simulink model "MCVT_Integration"  (R2020a)
settings: Solver=ode8  StartTime=0.0  StopTime=T_end  FixedStep=dt  RelTol=1e-3  EnableMultiTasking=on
values from neighbouring files (symbols this model uses):
  assumed_power_voltage = 1.25e2    (= 125)    [MCVT_Input_File.m]    (also in MCVT_Run_QuickCheck.m)
  battery_power_toggle = 1    [MCVT_Input_File.m]    (also in MCVT_Run_QuickCheck.m)
  battery_trickle_charge_param = 0.1    [MCVT_Input_File.m]    (also in MCVT_Run_QuickCheck.m)
  battery_trickle_leakage_param = 0.01    [MCVT_Input_File.m]    (also in MCVT_Run_QuickCheck.m)
  damage_case_id = 3    [MCVT_Input_File.m]    (also in MCVT_Run_QuickCheck.m = 1)
  dt = 1/fs    (= 0.001)    [MCVT_Run_QuickCheck.m]    (also in MCVT_Sim_Set.m)
  dt_2 = 1/fs_2    (= 0.001)    [MCVT_Run_QuickCheck.m]    (also in MCVT_Sim_Set.m)
  dt_3 = 1/fs_3    (= 0.001)    [MCVT_Run_QuickCheck.m]    (also in MCVT_Sim_Set.m)
  dt_5 = 1/fs_5    (= 0.005)    [MCVT_Run_QuickCheck.m]    (also in MCVT_Sim_Set.m)
  dt_8 = 1/fs_8    (= 0.05)    [MCVT_Run_QuickCheck.m]    (also in MCVT_Sim_Set.m)
  dt_9 = 1/fs_9    (= 0.001)    [MCVT_Run_QuickCheck.m]    (also in MCVT_Sim_Set.m)
  dust_met_duration = 3    [MCVT_Input_File.m]    (also in MCVT_Run_QuickCheck.m = 2)
  ECLSS_indoor_set_temperature = 298.15    [MCVT_Input_File.m]    (also in MCVT_Run_QuickCheck.m)
  energy_store_max_o = 150    [MCVT_Input_File.m]    (also in MCVT_Run_QuickCheck.m)
  energy_store_o = 120    [MCVT_Input_File.m]    (also in MCVT_Run_QuickCheck.m)
  fs = 1000    [MCVT_Run_QuickCheck.m]    (also in MCVT_Sim_Set.m)
  gain_charge_param = 1    [MCVT_Input_File.m]    (also in MCVT_Run_QuickCheck.m)
  ground_temperature = 373    [MCVT_Habitat_Design_Input_File.m]    (also in MCVT_Run_QuickCheck.m)
  I_max_charge = 800    [MCVT_Input_File.m]    (also in MCVT_Run_QuickCheck.m)
  I_max_discharge = 800    [MCVT_Input_File.m]    (also in MCVT_Run_QuickCheck.m)
  initial_pressure = 1e5    (= 100000)    [MCVT_Input_File.m]    (also in MCVT_Run_QuickCheck.m)
  initial_temperature = 295    [MCVT_Input_File.m]    (also in MCVT_Run_QuickCheck.m)
  interior_leak_rate = 0.00415    [MCVT_Input_File.m]    (also in MCVT_Run_QuickCheck.m)
  launch_dust_accum_start_time = 10    [MCVT_Input_File.m]    (also in MCVT_Run_QuickCheck.m = 2)
  launch_dust_accum_stop_time = 12    [MCVT_Input_File.m]    (also in MCVT_Run_QuickCheck.m = 3)
  meteorite_impact_time = 2    [MCVT_Input_File.m]    (also in MCVT_Run_QuickCheck.m = 1)
  nuclear_power_toggle = 1    [MCVT_Input_File.m]    (also in MCVT_Run_QuickCheck.m)
  print_interval = 30    [MCVT_Input_File.m]
  SOC_lower_thres = 0.5    [MCVT_Input_File.m]    (also in MCVT_Run_QuickCheck.m)
  SOC_upper_thres = 0.9    [MCVT_Input_File.m]    (also in MCVT_Run_QuickCheck.m)
  solar_power_toggle = 1    [MCVT_Input_File.m]    (also in MCVT_Run_QuickCheck.m)
  space_temperature = 4    [MCVT_Habitat_Design_Input_File.m]    (also in MCVT_Run_QuickCheck.m)
  Start_angle = 90    [MCVT_Input_File.m]    (also in MCVT_Run_QuickCheck.m)
undefined symbols (not defined in the file or in its folder): A_flow_restrictor, ACTIVITY_LOOKUP_TABLE, air, Aref, Bmap, Bmap2, Bmap3, comms, DiscIntNeverNeededParam, DmgSPL, dust_parameters, ECLSS_dust_damage_repair_time, ECLSS_heater_capacity, ECLSS_IPS_restrictor, ECLSS_IPS_setpointPressure, ECLSS_paint_damage_repair_time, ECLSS_radiatorLoop_discreteNumber, ECLSS_Vst, emptyActivity, expected_heat_add, FI, habitat, HIEM2SMM, HIEM2STM, HIEMDamageElements, impact_numbering, initial_SPLT_damage_index, input_air, input_Cond, input_CP, input_discretization, input_EV, input_Evap, input_radiatorLoop, K_ECLSS_dust_single, K_ECLSS_paint_single, K_Structure_damage_single, Kref, kW_to_W, meteoroidDustDep, mFRF, ndelays, nominalDustDep, noverlap, nuclear_toggle_gain, output_limits, panel_paint0, Ps0, reference_temperature, RepairTime_ECLSS_factor, Sensor_Location_SMM, SMM_T0, SMM_T1, SMM_T2, SMM_T3, SMM_T4, SMM_T4_0, SMM_T4_1, SMM_T4_2, solar_FDD_K ... (+73)
libraries: neural x350, simulink x39, xpcutilitieslib x7, xpcrtudplib x7, dspstat3 x1
(part 5 of 43)

== system MCVT_Integration/Sys3 - Power System/Power Generation Subsystem1/Power Distribution and Storage System/Subsystem2 ==
  Sub3_Power_Consumed_by_Sub2 (kW) : Inport
  Sub3_Power_Consumed_by_Sub5 (kW) : Inport  Port=2
  Sub3_Power_Consumed_by_Sub8 (kW) : Inport  Port=3
  2 : Memory  InitialCondition=50
  5 : Memory  InitialCondition=50
  Bus Creator1 : BusCreator  Inputs=3
  Dummy Electrical Power Consuming Equipment3 : Subsystem
  Sub3_Power_Concumption (kW) : Outport
  wires:
  Sub3_Power_Consumed_by_Sub5 (kW)/1 -> 5/1
  Sub3_Power_Consumed_by_Sub2 (kW)/1 -> 2/1
  Dummy Electrical Power Consuming Equipment3/1(p_3_desired) -> Bus Creator1/3  "p_3_desired"
  Bus Creator1/1 -> Sub3_Power_Concumption (kW)/1
  Sub3_Power_Consumed_by_Sub8 (kW)/1 -> Dummy Electrical Power Consuming Equipment3/1(XX_In_Power_Available)
  5/1 -> Bus Creator1/2  "p_2_desired"
  2/1 -> Bus Creator1/1  "p_1_desired"

== system MCVT_Integration/Sys5 - ECLSS/Active Thermal Control System (ATCS)/Faulty Panels/Sim-FDD-ECLSS-Dust ==
  xtrueHS : Inport
  EnableResetFDD_Dust : Inport  Port=2
  MATLAB Function2 : MATLAB Function  TreatAsAtomicUnit=on
  MATLAB System : MATLABSystem  System=ECLSS_simu_FDD_dust  timestep=[dt_5]  Ksingle=[K_ECLSS_dust_single]  noise=1e-2  num=50  SimulateUsing=Code generation
      mask Type: ECLSS_simu_FDD_dust
      mask Display:
      | disp('ECLSS_simu_FDD_dust');
      | port_label('input',1,'xtrue');
      | port_label('input',2,'EnableReset');
      | port_label('output',1,'xe');
  Scope : Scope  NumInputPorts=2
  FDD_ECLSS_dust_xe : Outport
  wires:
  EnableResetFDD_Dust/1 -> Scope/2, MATLAB System/2
  xtrueHS/1 -> MATLAB Function2/1(x)
  MATLAB Function2/1(y) -> MATLAB System/1
  MATLAB System/1 -> Scope/1, FDD_ECLSS_dust_xe/1

== system MCVT_Integration/Sys5 - ECLSS/Active Thermal Control System (ATCS)/Faulty Panels/Sim-FDD-ECLSS-Paint ==
  xtrueHS : Inport
  EnableResetFDD_Paint : Inport  Port=2
  MATLAB Function6 : MATLAB Function  TreatAsAtomicUnit=on
  MATLAB System1 : MATLABSystem  System=ECLSS_simu_FDD_paint  timestep=[dt_5]  Ksingle=[K_ECLSS_paint_single]  noise=1e-2  num=50  SimulateUsing=Code generation
      mask Type: ECLSS_simu_FDD_paint
      mask Display:
      | disp('ECLSS_simu_FDD_paint');
      | port_label('input',1,'xtrue');
      | port_label('input',2,'EnableReset');
      | port_label('output',1,'xe');
  FDD_ECLSS_paint_xe : Outport
  wires:
  MATLAB Function6/1(y) -> MATLAB System1/1
  EnableResetFDD_Paint/1 -> MATLAB System1/2
  MATLAB System1/1 -> FDD_ECLSS_paint_xe/1
  xtrueHS/1 -> MATLAB Function6/1(x)

== system MCVT_Integration/Sys8 - Interior Environment/Habitat Interior Environment Model/Temperature Sensors/ Resistance Temperature Detector ==
  Physical Interdependency - IN : Inport
  Switch Control : Inport  Port=2
  Power_Supply (kW) : Inport  Port=3
  Power Consumption : MATLAB Function  TreatAsAtomicUnit=on
  R = U/I : Gain  Gain=1/0.001
  R vs T : MATLAB Function  TreatAsAtomicUnit=on
  RTD with Self-heat Error : Subsystem
  RTD without Self-heat Error : Subsystem
  Self-heat Calculation : Subsystem
  Switch ON/OFF Control Block : Subsystem
  T vs R : MATLAB Function  TreatAsAtomicUnit=on
  Physical Interdependency - OUT : Outport  VectorParamsAs1DForOutWhenUnconnected=off
  Cyber Interdependency - OUT : Outport  Port=2  VectorParamsAs1DForOutWhenUnconnected=off
  Power_Usage (kW) : Outport  Port=3
  wires:
  T vs R/1(R) -> RTD without Self-heat Error/1(Resistance Change without Error), Self-heat Calculation/2(Resistance Change without Error)
  R vs T/1(T) -> Cyber Interdependency - OUT/1
  RTD with Self-heat Error/1(Voltage Output with Error) -> R = U/I/1
  Self-heat Calculation/1(Resistance Change with Error) -> RTD with Self-heat Error/1(Resistance Change with Error)
  R = U/I/1 -> R vs T/1(R)
  RTD without Self-heat Error/1(Current in Circuit) -> Self-heat Calculation/3(Current in Circuit)
  Switch ON/OFF Control Block/1(Out1) -> Power Consumption/2(Switch), T vs R/1(T), Self-heat Calculation/1(Real Temperature)
  Switch Control/1 -> Switch ON/OFF Control Block/1(In1)
  Physical Interdependency - IN/1 -> Physical Interdependency - OUT/1, Switch ON/OFF Control Block/2(In2)
  Power_Supply (kW)/1 -> Power Consumption/1(Supply)
  Power Consumption/1(kWUsage1) -> Power_Usage (kW)/1

== system MCVT_Integration/Sys8 - Interior Environment/Habitat Interior Environment Model/Temperature Sensors/ Resistance Temperature Detector1 ==
  Physical Interdependency - IN : Inport
  Switch Control : Inport  Port=2
  Power_Supply (kW) : Inport  Port=3
  Power Consumption : MATLAB Function  TreatAsAtomicUnit=on
  R = U/I : Gain  Gain=1/0.001
  R vs T : MATLAB Function  TreatAsAtomicUnit=on
  RTD with Self-heat Error : Subsystem
  RTD without Self-heat Error : Subsystem
  Self-heat Calculation : Subsystem
  Switch ON/OFF Control Block : Subsystem
  T vs R : MATLAB Function  TreatAsAtomicUnit=on
  Physical Interdependency - OUT : Outport  VectorParamsAs1DForOutWhenUnconnected=off
  Cyber Interdependency - OUT : Outport  Port=2  VectorParamsAs1DForOutWhenUnconnected=off
  Power_Usage (kW) : Outport  Port=3
  wires:
  Physical Interdependency - IN/1 -> Switch ON/OFF Control Block/2(In2), Physical Interdependency - OUT/1
  Switch Control/1 -> Switch ON/OFF Control Block/1(In1)
  RTD without Self-heat Error/1(Current in Circuit) -> Self-heat Calculation/3(Current in Circuit)
  R = U/I/1 -> R vs T/1(R)
  Self-heat Calculation/1(Resistance Change with Error) -> RTD with Self-heat Error/1(Resistance Change with Error)
  RTD with Self-heat Error/1(Voltage Output with Error) -> R = U/I/1
  R vs T/1(T) -> Cyber Interdependency - OUT/1
  T vs R/1(R) -> Self-heat Calculation/2(Resistance Change without Error), RTD without Self-heat Error/1(Resistance Change without Error)
  Power Consumption/1(kWUsage2) -> Power_Usage (kW)/1
  Switch ON/OFF Control Block/1(Out1) -> Power Consumption/2(Switch), Self-heat Calculation/1(Real Temperature), T vs R/1(T)
  Power_Supply (kW)/1 -> Power Consumption/1(Supply)

== system MCVT_Integration/Sys8 - Interior Environment/Habitat Interior Environment Model/Temperature Sensors/ Resistance Temperature Detector2 ==
  Physical Interdependency - IN : Inport
  Switch Control : Inport  Port=2
  Power_Supply (kW) : Inport  Port=3
  Power Consumption : MATLAB Function  TreatAsAtomicUnit=on
  R = U/I : Gain  Gain=1/0.001
  R vs T : MATLAB Function  TreatAsAtomicUnit=on
  RTD with Self-heat Error : Subsystem
  RTD without Self-heat Error : Subsystem
  Self-heat Calculation : Subsystem
  Switch ON/OFF Control Block : Subsystem
  T vs R : MATLAB Function  TreatAsAtomicUnit=on
  Physical Interdependency - OUT : Outport  VectorParamsAs1DForOutWhenUnconnected=off
  Cyber Interdependency - OUT : Outport  Port=2  VectorParamsAs1DForOutWhenUnconnected=off
  Power_Usage (kW) : Outport  Port=3
  wires:
  T vs R/1(R) -> RTD without Self-heat Error/1(Resistance Change without Error), Self-heat Calculation/2(Resistance Change without Error)
  R vs T/1(T) -> Cyber Interdependency - OUT/1
  RTD with Self-heat Error/1(Voltage Output with Error) -> R = U/I/1
  Self-heat Calculation/1(Resistance Change with Error) -> RTD with Self-heat Error/1(Resistance Change with Error)
  R = U/I/1 -> R vs T/1(R)
  RTD without Self-heat Error/1(Current in Circuit) -> Self-heat Calculation/3(Current in Circuit)
  Switch Control/1 -> Switch ON/OFF Control Block/1(In1)
  Physical Interdependency - IN/1 -> Switch ON/OFF Control Block/2(In2), Physical Interdependency - OUT/1
  Power Consumption/1(kWUsage3) -> Power_Usage (kW)/1
  Switch ON/OFF Control Block/1(Out1) -> Power Consumption/2(Switch), T vs R/1(T), Self-heat Calculation/1(Real Temperature)
  Power_Supply (kW)/1 -> Power Consumption/1(Supply)

== system MCVT_Integration/Sys 6 - Health Management and Agents/Decision Maker/Simulink Communication Modules/Agent//MCVT API from HMS/UDP_Receive_C2 ==
  "Byte Unpacking " : xpcutilitieslib/Byte Unpacking   MaskUnpackedDataTypes={'uint8','uint16','double'}  MaskUnpackedDataSizes={[6],[1],[comms.recv.Agent.nD1]}  MaskAlignment=1
      settings: SourceProductBaseCode=XP
  Data Type Conversion : DataTypeConversion  OutDataTypeStr=double
  Mux : Mux  Inputs=2
  Receive : xpcrtudplib/UDP Receive  ipAddressVal=Use host-target connection  localPort=comms.recv.Agent.LPORT  rcvWidth=comms.recv.Agent.nD1*8+8  rcvFmAny=off  fmAddress=comms.Remote_IP  rcvMulticast=off  multicastAddress={}  maxUDPQueue=8  sampleTime=dt_2
  Terminator : Terminator
  Receive_file : Outport
  Receive_output : Outport  Port=2
  Receive_length : Outport  Port=3
  wires:
  Receive/1 -> "Byte Unpacking "/1
  "Byte Unpacking "/3 -> Mux/1, Receive_output/1
  "Byte Unpacking "/1 -> Terminator/1
  Receive/2 -> Receive_length/1
  Mux/1 -> Receive_file/1
  "Byte Unpacking "/2 -> Data Type Conversion/1
  Data Type Conversion/1 -> Mux/2

== system MCVT_Integration/Sys 6 - Health Management and Agents/Decision Maker/Simulink Communication Modules/Agent//MCVT API to HMS/UDP_Send_C2 ==
  Input_Send : Inport
  Packet_Info : Inport  Port=2
  "Byte Packing " : xpcutilitieslib/Byte Packing   MaskPackedDataType=uint8  MaskUnpackedDataTypes={'uint8','uint16','double'}  MaskAlignment=1
  Data Type Conversion : DataTypeConversion  OutDataTypeStr=uint8
  Data Type Conversion1 : DataTypeConversion  OutDataTypeStr=uint16
  MATLAB Function : MATLAB Function  TreatAsAtomicUnit=on
  MATLAB Function1 : MATLAB Function  TreatAsAtomicUnit=on
  Send1 : xpcrtudplib/UDP Send  ipAddressVal=Use host-target connection  localPort=comms.send.Agent.LPORT  toAddress=comms.Remote_IP  toPort=comms.send.Agent.TOPORT  sampleTime=comms.dt_NRT_comm
      settings: SourceProductBaseCode=XP
  Width : Width
  Width1 : Width
  Send_file : Outport
  wires:
  Width1/1 -> MATLAB Function/1(len)
  Input_Send/1 -> Send_file/1, "Byte Packing "/3, Width1/1, MATLAB Function1/1(signal)
  "Byte Packing "/1 -> Width/1, Send1/1
  Width/1 -> Send1/2
  MATLAB Function/1(hi0) -> Data Type Conversion/1
  Data Type Conversion/1 -> "Byte Packing "/1
  Packet_Info/1 -> MATLAB Function/2(hdr_Info)
  MATLAB Function1/1(SizeInfo) -> MATLAB Function/3(rowcol)
  MATLAB Function/2(len0) -> Data Type Conversion1/1
  Data Type Conversion1/1 -> "Byte Packing "/2

== system MCVT_Integration/Sys 6 - Health Management and Agents/Decision Maker/Simulink Communication Modules/ECLSS_Dust//MCVT API to HMS/UDP_Send ==
  Input_Send : Inport
  Packet_Info : Inport  Port=2
  "Byte Packing " : xpcutilitieslib/Byte Packing   MaskPackedDataType=uint8  MaskUnpackedDataTypes={'uint8','uint16','double'}  MaskAlignment=1
  Data Type Conversion : DataTypeConversion  OutDataTypeStr=uint8
  Data Type Conversion1 : DataTypeConversion  OutDataTypeStr=uint16
  MATLAB Function : MATLAB Function  TreatAsAtomicUnit=on
  MATLAB Function1 : MATLAB Function  TreatAsAtomicUnit=on
  Send1 : xpcrtudplib/UDP Send  ipAddressVal=Use host-target connection  localPort=comms.send.ECLSS.FDD_Dust.LPORT  toAddress=comms.Remote_IP  toPort=comms.send.ECLSS.FDD_Dust.TOPORT  sampleTime=comms.dt_NRT_comm
      settings: SourceProductBaseCode=XP
  Width : Width
  Width1 : Width
  Send_file : Outport
  wires:
  Width1/1 -> MATLAB Function/1(len)
  Input_Send/1 -> Send_file/1, "Byte Packing "/3, Width1/1, MATLAB Function1/1(signal)
  "Byte Packing "/1 -> Width/1, Send1/1
  Width/1 -> Send1/2
  MATLAB Function/1(hi0) -> Data Type Conversion/1
  Data Type Conversion/1 -> "Byte Packing "/1
  MATLAB Function1/1(SizeInfo) -> MATLAB Function/3(rowcol)
  MATLAB Function/2(len0) -> Data Type Conversion1/1
  Data Type Conversion1/1 -> "Byte Packing "/2
  Packet_Info/1 -> MATLAB Function/2(hdr_Info)

== system MCVT_Integration/Sys 6 - Health Management and Agents/Decision Maker/Simulink Communication Modules/ECLSS_Paint//MCVT API to HMS/UDP_Send ==
  Input_Send : Inport
  Packet_Info : Inport  Port=2
  "Byte Packing " : xpcutilitieslib/Byte Packing   MaskPackedDataType=uint8  MaskUnpackedDataTypes={'uint8','uint16','double'}  MaskAlignment=1
  Data Type Conversion : DataTypeConversion  OutDataTypeStr=uint8
  Data Type Conversion1 : DataTypeConversion  OutDataTypeStr=uint16
  MATLAB Function : MATLAB Function  TreatAsAtomicUnit=on
  MATLAB Function1 : MATLAB Function  TreatAsAtomicUnit=on
  Send1 : xpcrtudplib/UDP Send  ipAddressVal=Use host-target connection  localPort=comms.send.ECLSS.FDD_Paint.LPORT  toAddress=comms.Remote_IP  toPort=comms.send.ECLSS.FDD_Paint.TOPORT  sampleTime=comms.dt_NRT_comm
      settings: SourceProductBaseCode=XP
  Width : Width
  Width1 : Width
  Send_file : Outport
  wires:
  Width1/1 -> MATLAB Function/1(len)
  Input_Send/1 -> Send_file/1, "Byte Packing "/3, Width1/1, MATLAB Function1/1(signal)
  "Byte Packing "/1 -> Width/1, Send1/1
  Width/1 -> Send1/2
  MATLAB Function/1(hi0) -> Data Type Conversion/1
  Data Type Conversion/1 -> "Byte Packing "/1
  Packet_Info/1 -> MATLAB Function/2(hdr_Info)
  MATLAB Function1/1(SizeInfo) -> MATLAB Function/3(rowcol)
  MATLAB Function/2(len0) -> Data Type Conversion1/1
  Data Type Conversion1/1 -> "Byte Packing "/2

== system MCVT_Integration/Sys 6 - Health Management and Agents/Decision Maker/Simulink Communication Modules/NPG_Dust//MCVT API to HMS/UDP_Send ==
  Input_Send : Inport
  Packet_Info : Inport  Port=2
  "Byte Packing " : xpcutilitieslib/Byte Packing   MaskPackedDataType=uint8  MaskUnpackedDataTypes={'uint8','uint16','double'}  MaskAlignment=1
  Data Type Conversion : DataTypeConversion  OutDataTypeStr=uint8
  Data Type Conversion1 : DataTypeConversion  OutDataTypeStr=uint16
  MATLAB Function : MATLAB Function  TreatAsAtomicUnit=on
  MATLAB Function1 : MATLAB Function  TreatAsAtomicUnit=on
  Send1 : xpcrtudplib/UDP Send  ipAddressVal=Use host-target connection  localPort=comms.send.NPG.FDD_Dust.LPORT  toAddress=comms.Remote_IP  toPort=comms.send.NPG.FDD_Dust.TOPORT  sampleTime=comms.dt_NRT_comm
      settings: SourceProductBaseCode=XP
  Width : Width
  Width1 : Width
  Send_file : Outport
  wires:
  Width1/1 -> MATLAB Function/1(len)
  Input_Send/1 -> Send_file/1, "Byte Packing "/3, Width1/1, MATLAB Function1/1(signal)
  "Byte Packing "/1 -> Width/1, Send1/1
  Width/1 -> Send1/2
  MATLAB Function/1(hi0) -> Data Type Conversion/1
  Data Type Conversion/1 -> "Byte Packing "/1
  Packet_Info/1 -> MATLAB Function/2(hdr_Info)
  MATLAB Function1/1(SizeInfo) -> MATLAB Function/3(rowcol)
  MATLAB Function/2(len0) -> Data Type Conversion1/1
  Data Type Conversion1/1 -> "Byte Packing "/2

== system MCVT_Integration/Sys 6 - Health Management and Agents/Decision Maker/Simulink Communication Modules/Solar_PV//MCVT API to HMS/UDP_Send ==
  Input_Send : Inport
  Packet_Info : Inport  Port=2
  "Byte Packing " : xpcutilitieslib/Byte Packing   MaskPackedDataType=uint8  MaskUnpackedDataTypes={'uint8','uint16','double'}  MaskAlignment=1
  Data Type Conversion : DataTypeConversion  OutDataTypeStr=uint8
  Data Type Conversion1 : DataTypeConversion  OutDataTypeStr=uint16
  MATLAB Function : MATLAB Function  TreatAsAtomicUnit=on
  MATLAB Function1 : MATLAB Function  TreatAsAtomicUnit=on
  Send1 : xpcrtudplib/UDP Send  ipAddressVal=Use host-target connection  localPort=comms.send.SPG.FDD_Dust.LPORT  toAddress=comms.Remote_IP  toPort=comms.send.SPG.FDD_Dust.TOPORT  sampleTime=comms.dt_NRT_comm
      settings: SourceProductBaseCode=XP
  Width : Width
  Width1 : Width
  Send_file : Outport
  wires:
  Width1/1 -> MATLAB Function/1(len)
  Input_Send/1 -> Send_file/1, "Byte Packing "/3, Width1/1, MATLAB Function1/1(signal)
  "Byte Packing "/1 -> Width/1, Send1/1
  Width/1 -> Send1/2
  MATLAB Function/1(hi0) -> Data Type Conversion/1
  Data Type Conversion/1 -> "Byte Packing "/1
  Packet_Info/1 -> MATLAB Function/2(hdr_Info)
  MATLAB Function1/1(SizeInfo) -> MATLAB Function/3(rowcol)
  MATLAB Function/2(len0) -> Data Type Conversion1/1
  Data Type Conversion1/1 -> "Byte Packing "/2

== system MCVT_Integration/Sys 6 - Health Management and Agents/Decision Maker/Simulink Communication Modules/Structure_Dmg//MCVT API to HMS/UDP_Send ==
  Input_Send : Inport
  Packet_Info : Inport  Port=2
  "Byte Packing " : xpcutilitieslib/Byte Packing   MaskPackedDataType=uint8  MaskUnpackedDataTypes={'uint8','uint16','double'}  MaskAlignment=1
  Data Type Conversion : DataTypeConversion  OutDataTypeStr=uint8
  Data Type Conversion1 : DataTypeConversion  OutDataTypeStr=uint16
  MATLAB Function : MATLAB Function  TreatAsAtomicUnit=on
  MATLAB Function1 : MATLAB Function  TreatAsAtomicUnit=on
  Send1 : xpcrtudplib/UDP Send  ipAddressVal=Use host-target connection  localPort=comms.send.Str.FDD_Dmg.LPORT  toAddress=comms.Remote_IP  toPort=comms.send.Str.FDD_Dmg.TOPORT  sampleTime=comms.dt_NRT_comm
      settings: SourceProductBaseCode=XP
  Width : Width
  Width1 : Width
  Send_file : Outport
  wires:
  Width1/1 -> MATLAB Function/1(len)
  Input_Send/1 -> Send_file/1, "Byte Packing "/3, Width1/1, MATLAB Function1/1(signal)
  "Byte Packing "/1 -> Width/1, Send1/1
  Width/1 -> Send1/2
  MATLAB Function/1(hi0) -> Data Type Conversion/1
  Data Type Conversion/1 -> "Byte Packing "/1
  Packet_Info/1 -> MATLAB Function/2(hdr_Info)
  MATLAB Function1/1(SizeInfo) -> MATLAB Function/3(rowcol)
  MATLAB Function/2(len0) -> Data Type Conversion1/1
  Data Type Conversion1/1 -> "Byte Packing "/2

== system MCVT_Integration/Sys2 - Structural System/Sys2_Structural_System/Fault Detection and Diagnosis (FDD) 2.3/Accelerometer/Acceleration Delay ==
  Measured Acceleration : Inport
  Matrix Concatenate : Concatenate  NumInputs=9  Mode=Multidimensional array  ConcatenateDimension=2
  Selector1 : Selector  InputPortWidth=9  IndexOptions=Index vector (dialog)  Indices=[1]  OutputSizes=1
  Selector2 : Selector  InputPortWidth=9  IndexOptions=Index vector (dialog)  Indices=[2]  OutputSizes=1
  Selector3 : Selector  InputPortWidth=9  IndexOptions=Index vector (dialog)  Indices=[3]  OutputSizes=1
  Selector4 : Selector  InputPortWidth=9  IndexOptions=Index vector (dialog)  Indices=[4]  OutputSizes=1
  Selector5 : Selector  InputPortWidth=9  IndexOptions=Index vector (dialog)  Indices=[5]  OutputSizes=1
  Selector6 : Selector  InputPortWidth=9  IndexOptions=Index vector (dialog)  Indices=[6]  OutputSizes=1
  Selector7 : Selector  InputPortWidth=9  IndexOptions=Index vector (dialog)  Indices=[7]  OutputSizes=1
  Selector8 : Selector  InputPortWidth=9  IndexOptions=Index vector (dialog)  Indices=[8]  OutputSizes=1
  Selector9 : Selector  InputPortWidth=9  IndexOptions=Index vector (dialog)  Indices=[9]  OutputSizes=1
  Tapped Delay {1..9} (9 alike) : simulink/Discrete/Tapped Delay  vinit=0.0  samptime=-1  NumDelays=ndelays  DelayOrder=Oldest  includeCurrent=off
  Delayed Acceleration : Outport
  wires:
  Tapped Delay 9/1 -> Matrix Concatenate/9
  Tapped Delay 8/1 -> Matrix Concatenate/8
  Tapped Delay 7/1 -> Matrix Concatenate/7
  Tapped Delay 6/1 -> Matrix Concatenate/6
  Tapped Delay 5/1 -> Matrix Concatenate/5
  Tapped Delay 4/1 -> Matrix Concatenate/4
  Tapped Delay 3/1 -> Matrix Concatenate/3
  Tapped Delay 2/1 -> Matrix Concatenate/2
  Tapped Delay 1/1 -> Matrix Concatenate/1
  Measured Acceleration/1 -> Selector1/1, Selector2/1, Selector3/1, Selector4/1, Selector5/1, Selector6/1, Selector7/1, Selector8/1, Selector9/1
  Selector2/1 -> Tapped Delay 2/1  "Actural Acceleration"
  Selector1/1 -> Tapped Delay 1/1  "Actural Acceleration"
  Selector7/1 -> Tapped Delay 7/1  "Actural Acceleration"
  Selector6/1 -> Tapped Delay 6/1  "Actural Acceleration"
  Selector4/1 -> Tapped Delay 4/1  "Actural Acceleration"
  Selector5/1 -> Tapped Delay 5/1  "Actural Acceleration"
  Selector3/1 -> Tapped Delay 3/1  "Actural Acceleration"
  Matrix Concatenate/1 -> Delayed Acceleration/1
  Selector9/1 -> Tapped Delay 9/1  "Actural Acceleration"
  Selector8/1 -> Tapped Delay 8/1  "Actural Acceleration"

== system MCVT_Integration/Sys2 - Structural System/Sys2_Structural_System/Fault Detection and Diagnosis (FDD) 2.3/Accelerometer/Accelerometer ==
  note: 1 -- Turn sensors on
      | 2 -- Turn sesnors off
  note: Damage States
      | State 1 - Healthy
      | State 2 - Partially Damaged1: Amplitude Change
      | State 3 - Partially Damaged2: Data Loss
      | State 4 - Failed
  Physical Interdependency - IN : Inport
  SensorPowerSupply : Inport  Port=2
  " Switch ON/OFF Control Number" : MATLAB Function  TreatAsAtomicUnit=on
  Accelerometer : Subsystem
  Bias Voltage : Constant  Value=12
  Clock : Clock
  Damage State Control Block : MATLAB Function  TreatAsAtomicUnit=on
  Damage State Control Number : Constant  Value=[1;1;1]
  Minus : Sum  Inputs=+-
  "Sensitivity " : Gain  Gain=1000
  Sensor Number : Constant  Value=[1;2;3]
  Switch ON/OFF Control Block : Subsystem
  Cyber Interdependency - OUT : Outport  VectorParamsAs1DForOutWhenUnconnected=off
  wires:
  "Sensitivity "/1 -> Cyber Interdependency - OUT/1
  Accelerometer/1(Out) -> Damage State Control Block/2(Acc)
  Damage State Control Block/1(y) -> Minus/1
  Damage State Control Number/1 -> Damage State Control Block/1(state_number)
  Clock/1 -> Damage State Control Block/3(Time)
  Sensor Number/1 -> Switch ON/OFF Control Block/2(In2)
  Switch ON/OFF Control Block/1(Out1) -> Accelerometer/1(In1)
  Physical Interdependency - IN/1 -> Switch ON/OFF Control Block/3(In3)
  Bias Voltage/1 -> Minus/2
  Minus/1 -> "Sensitivity "/1
  SensorPowerSupply/1 -> " Switch ON/OFF Control Number"/1(p)
  " Switch ON/OFF Control Number"/1(y) -> Switch ON/OFF Control Block/1(In1)

== system MCVT_Integration/Sys2 - Structural System/Sys2_Structural_System/Fault Detection and Diagnosis (FDD) 2.3/Pattern Recognition Neural Network/Pattern Recognition Neural Network with 10% Noise ==
  Input : Inport  PortDimensions=2304
      mask Display: e = 0.06;c1 = [120 230 180]/255;c2 = ([120 230 180]+0)/(256+128);c3 = ([120 230 180]+128)/(256+128);patch([0 1 1 0],[0 0 1 1],c1);patch([0 1 1 1-e 1-e 0+e],[0 0 1 1-e 0+e 0+e],c2);patch([0 0 1 1-e 0+e 0+e],[0 1 1 1-e 1-e 0+e],c3);plot([0 1 1 0 0],[0 0 1 1 0]);
  Layer 1 : Subsystem
      mask Display: e = 0.06;c1 = [120 150 230]/255;c2 = ([120 150 230]+0)/383;c3 = ([120 150 230]+128)/383;patch([0 1 1 0],[0 0 1 1],c1);patch([0 1 1 1-e 1-e 0+e],[0 0 1 1-e 0+e 0+e],c2);patch([0 0 1 1-e 0+e 0+e],[0 1 1 1-e 1-e 0+e],c3);plot([0 1 1 0 0],[0 0 1 1 0]);
  Layer 2 : Subsystem
      mask Display: e = 0.06;c1 = [120 150 230]/255;c2 = ([120 150 230]+0)/383;c3 = ([120 150 230]+128)/383;patch([0 1 1 0],[0 0 1 1],c1);patch([0 1 1 1-e 1-e 0+e],[0 0 1 1-e 0+e 0+e],c2);patch([0 0 1 1-e 0+e 0+e],[0 1 1 1-e 1-e 0+e],c3);plot([0 1 1 0 0],[0 0 1 1 0]);
  Layer 3 : Subsystem
      mask Display: e = 0.06;c1 = [120 150 230]/255;c2 = ([120 150 230]+0)/383;c3 = ([120 150 230]+128)/383;patch([0 1 1 0],[0 0 1 1],c1);patch([0 1 1 1-e 1-e 0+e],[0 0 1 1-e 0+e 0+e],c2);patch([0 0 1 1-e 0+e 0+e],[0 1 1 1-e 1-e 0+e],c3);plot([0 1 1 0 0],[0 0 1 1 0]);
  Process Input 1 : Subsystem
      mask Display: e = 0.06;c1 = [120 150 230]/255;c2 = ([120 150 230]+0)/383;c3 = ([120 150 230]+128)/383;patch([0 1 1 0],[0 0 1 1],c1);patch([0 1 1 1-e 1-e 0+e],[0 0 1 1-e 0+e 0+e],c2);patch([0 0 1 1-e 0+e 0+e],[0 1 1 1-e 1-e 0+e],c3);plot([0 1 1 0 0],[0 0 1 1 0]);
  Process Output 1 : Subsystem
      mask Display: e = 0.06;c1 = [120 150 230]/255;c2 = ([120 150 230]+0)/383;c3 = ([120 150 230]+128)/383;patch([0 1 1 0],[0 0 1 1],c1);patch([0 1 1 1-e 1-e 0+e],[0 0 1 1-e 0+e 0+e],c2);patch([0 0 1 1-e 0+e 0+e],[0 1 1 1-e 1-e 0+e],c3);plot([0 1 1 0 0],[0 0 1 1 0]);
  Output : Outport  InitialOutput=[0;0;0;0;0;0]
      mask Display: e = 0.06;c1 = [120 230 180]/255;c2 = ([120 230 180]+0)/(256+128);c3 = ([120 230 180]+128)/(256+128);patch([0 1 1 0],[0 0 1 1],c1);patch([0 1 1 1-e 1-e 0+e],[0 0 1 1-e 0+e 0+e],c2);patch([0 0 1 1-e 0+e 0+e],[0 1 1 1-e 1-e 0+e],c3);plot([0 1 1 0 0],[0 0 1 1 0]);
  wires:
  Input/1 -> Process Input 1/1(x)
  Process Output 1/1(y) -> Output/1
  Process Input 1/1(p) -> Layer 1/1("p{1}")
  Layer 1/1("a{1}") -> [feedback1]
  [feedback1] -> Layer 2/1("a{1} ")
  Layer 2/1("a{2}") -> [feedback2]
  [feedback2] -> Layer 3/1("a{2} ")
  Layer 3/1("a{3}") -> Process Output 1/1(a)

== system MCVT_Integration/Sys2 - Structural System/Sys2_Structural_System/Fault Detection and Diagnosis (FDD) with Binary Classifer/Accelerometer/Acceleration Delay ==
  Measured Acceleration : Inport
  Matrix Concatenate : Concatenate  NumInputs=9  Mode=Multidimensional array  ConcatenateDimension=2
  Selector1 : Selector  InputPortWidth=9  IndexOptions=Index vector (dialog)  Indices=[1]  OutputSizes=1
  Selector2 : Selector  InputPortWidth=9  IndexOptions=Index vector (dialog)  Indices=[2]  OutputSizes=1
  Selector3 : Selector  InputPortWidth=9  IndexOptions=Index vector (dialog)  Indices=[3]  OutputSizes=1
  Selector4 : Selector  InputPortWidth=9  IndexOptions=Index vector (dialog)  Indices=[4]  OutputSizes=1
  Selector5 : Selector  InputPortWidth=9  IndexOptions=Index vector (dialog)  Indices=[5]  OutputSizes=1
  Selector6 : Selector  InputPortWidth=9  IndexOptions=Index vector (dialog)  Indices=[6]  OutputSizes=1
  Selector7 : Selector  InputPortWidth=9  IndexOptions=Index vector (dialog)  Indices=[7]  OutputSizes=1
  Selector8 : Selector  InputPortWidth=9  IndexOptions=Index vector (dialog)  Indices=[8]  OutputSizes=1
  Selector9 : Selector  InputPortWidth=9  IndexOptions=Index vector (dialog)  Indices=[9]  OutputSizes=1
  Tapped Delay {1..9} (9 alike) : simulink/Discrete/Tapped Delay  vinit=0.0  samptime=-1  NumDelays=ndelays  DelayOrder=Oldest  includeCurrent=off
  Delayed Acceleration : Outport
  wires:
  Tapped Delay 9/1 -> Matrix Concatenate/9
  Tapped Delay 8/1 -> Matrix Concatenate/8
  Tapped Delay 7/1 -> Matrix Concatenate/7
  Tapped Delay 6/1 -> Matrix Concatenate/6
  Tapped Delay 5/1 -> Matrix Concatenate/5
  Tapped Delay 4/1 -> Matrix Concatenate/4
  Tapped Delay 3/1 -> Matrix Concatenate/3
  Tapped Delay 2/1 -> Matrix Concatenate/2
  Tapped Delay 1/1 -> Matrix Concatenate/1
  Measured Acceleration/1 -> Selector1/1, Selector2/1, Selector3/1, Selector4/1, Selector5/1, Selector6/1, Selector7/1, Selector8/1, Selector9/1
  Selector2/1 -> Tapped Delay 2/1  "Actural Acceleration"
  Selector1/1 -> Tapped Delay 1/1  "Actural Acceleration"
  Selector7/1 -> Tapped Delay 7/1  "Actural Acceleration"
  Selector6/1 -> Tapped Delay 6/1  "Actural Acceleration"
  Selector4/1 -> Tapped Delay 4/1  "Actural Acceleration"
  Selector5/1 -> Tapped Delay 5/1  "Actural Acceleration"
  Selector3/1 -> Tapped Delay 3/1  "Actural Acceleration"
  Matrix Concatenate/1 -> Delayed Acceleration/1
  Selector9/1 -> Tapped Delay 9/1  "Actural Acceleration"
  Selector8/1 -> Tapped Delay 8/1  "Actural Acceleration"

== system MCVT_Integration/Sys2 - Structural System/Sys2_Structural_System/Fault Detection and Diagnosis (FDD) with Binary Classifer/Accelerometer/Accelerometer ==
  note: 1 -- Turn sensors on
      | 2 -- Turn sesnors off
  note: Damage States
      | State 1 - Healthy
      | State 2 - Partially Damaged1: Amplitude Change
      | State 3 - Partially Damaged2: Data Loss
      | State 4 - Totally Failed
  Physical Interdependency - IN : Inport
  SensorPowerSupply : Inport  Port=2
  " Switch ON/OFF Control Number" : MATLAB Function  TreatAsAtomicUnit=on
  Accelerometer : Subsystem
  Actural Acc : Scope
  Bias Voltage : Constant  Value=12
  Clock : Clock
  Damage State Control Block : MATLAB Function  TreatAsAtomicUnit=on
  Damage State Control Number : Constant  Value=[1;1;1]
  Measured Acc : Scope
  Minus : Sum  Inputs=+-
  "Sensitivity " : Gain  Gain=1000
  Sensor Number : Constant  Value=[1;2;3]
  Switch ON/OFF Control Block : Subsystem
  Cyber Interdependency - OUT : Outport  VectorParamsAs1DForOutWhenUnconnected=off
  wires:
  "Sensitivity "/1 -> Cyber Interdependency - OUT/1, Measured Acc/1
  Accelerometer/1(Out) -> Damage State Control Block/2(Acc)
  Damage State Control Block/1(y) -> Minus/1
  Damage State Control Number/1 -> Damage State Control Block/1(state_number)
  Clock/1 -> Damage State Control Block/3(Time)
  Sensor Number/1 -> Switch ON/OFF Control Block/2(In2)
  Switch ON/OFF Control Block/1(Out1) -> Accelerometer/1(In1)
  Physical Interdependency - IN/1 -> Actural Acc/1, Switch ON/OFF Control Block/3(In3)
  Bias Voltage/1 -> Minus/2
  Minus/1 -> "Sensitivity "/1
  SensorPowerSupply/1 -> " Switch ON/OFF Control Number"/1(p)
  " Switch ON/OFF Control Number"/1(y) -> Switch ON/OFF Control Block/1(In1)

== system MCVT_Integration/Sys2 - Structural System/Sys2_Structural_System/Fault Detection and Diagnosis (FDD) with Binary Classifer/Pattern Recognition Neural Network/Segment 1 ==
  Input : Inport  PortDimensions=2304
      mask Display: e = 0.06;c1 = [120 230 180]/255;c2 = ([120 230 180]+0)/(256+128);c3 = ([120 230 180]+128)/(256+128);patch([0 1 1 0],[0 0 1 1],c1);patch([0 1 1 1-e 1-e 0+e],[0 0 1 1-e 0+e 0+e],c2);patch([0 0 1 1-e 0+e 0+e],[0 1 1 1-e 1-e 0+e],c3);plot([0 1 1 0 0],[0 0 1 1 0]);
  Layer 1 : Subsystem
      mask Display: e = 0.06;c1 = [120 150 230]/255;c2 = ([120 150 230]+0)/383;c3 = ([120 150 230]+128)/383;patch([0 1 1 0],[0 0 1 1],c1);patch([0 1 1 1-e 1-e 0+e],[0 0 1 1-e 0+e 0+e],c2);patch([0 0 1 1-e 0+e 0+e],[0 1 1 1-e 1-e 0+e],c3);plot([0 1 1 0 0],[0 0 1 1 0]);
  Layer 2 : Subsystem
      mask Display: e = 0.06;c1 = [120 150 230]/255;c2 = ([120 150 230]+0)/383;c3 = ([120 150 230]+128)/383;patch([0 1 1 0],[0 0 1 1],c1);patch([0 1 1 1-e 1-e 0+e],[0 0 1 1-e 0+e 0+e],c2);patch([0 0 1 1-e 0+e 0+e],[0 1 1 1-e 1-e 0+e],c3);plot([0 1 1 0 0],[0 0 1 1 0]);
  Layer 3 : Subsystem
      mask Display: e = 0.06;c1 = [120 150 230]/255;c2 = ([120 150 230]+0)/383;c3 = ([120 150 230]+128)/383;patch([0 1 1 0],[0 0 1 1],c1);patch([0 1 1 1-e 1-e 0+e],[0 0 1 1-e 0+e 0+e],c2);patch([0 0 1 1-e 0+e 0+e],[0 1 1 1-e 1-e 0+e],c3);plot([0 1 1 0 0],[0 0 1 1 0]);
  Process Input 1 : Subsystem
      mask Display: e = 0.06;c1 = [120 150 230]/255;c2 = ([120 150 230]+0)/383;c3 = ([120 150 230]+128)/383;patch([0 1 1 0],[0 0 1 1],c1);patch([0 1 1 1-e 1-e 0+e],[0 0 1 1-e 0+e 0+e],c2);patch([0 0 1 1-e 0+e 0+e],[0 1 1 1-e 1-e 0+e],c3);plot([0 1 1 0 0],[0 0 1 1 0]);
  Process Output 1 : Subsystem
      mask Display: e = 0.06;c1 = [120 150 230]/255;c2 = ([120 150 230]+0)/383;c3 = ([120 150 230]+128)/383;patch([0 1 1 0],[0 0 1 1],c1);patch([0 1 1 1-e 1-e 0+e],[0 0 1 1-e 0+e 0+e],c2);patch([0 0 1 1-e 0+e 0+e],[0 1 1 1-e 1-e 0+e],c3);plot([0 1 1 0 0],[0 0 1 1 0]);
  Output : Outport  InitialOutput=[0;0]
      mask Display: e = 0.06;c1 = [120 230 180]/255;c2 = ([120 230 180]+0)/(256+128);c3 = ([120 230 180]+128)/(256+128);patch([0 1 1 0],[0 0 1 1],c1);patch([0 1 1 1-e 1-e 0+e],[0 0 1 1-e 0+e 0+e],c2);patch([0 0 1 1-e 0+e 0+e],[0 1 1 1-e 1-e 0+e],c3);plot([0 1 1 0 0],[0 0 1 1 0]);
  wires:
  Input/1 -> Process Input 1/1(x)
  Process Output 1/1(y) -> Output/1
  Process Input 1/1(p) -> Layer 1/1("p{1}")
  Layer 1/1("a{1}") -> [feedback1]
  [feedback1] -> Layer 2/1("a{1} ")
  Layer 2/1("a{2}") -> [feedback2]
  [feedback2] -> Layer 3/1("a{2} ")
  Layer 3/1("a{3}") -> Process Output 1/1(a)

== system MCVT_Integration/Sys2 - Structural System/Sys2_Structural_System/Fault Detection and Diagnosis (FDD) with Binary Classifer/Pattern Recognition Neural Network/Segment 2 (2) ==
  Input : Inport  PortDimensions=2304
      mask Display: e = 0.06;c1 = [120 230 180]/255;c2 = ([120 230 180]+0)/(256+128);c3 = ([120 230 180]+128)/(256+128);patch([0 1 1 0],[0 0 1 1],c1);patch([0 1 1 1-e 1-e 0+e],[0 0 1 1-e 0+e 0+e],c2);patch([0 0 1 1-e 0+e 0+e],[0 1 1 1-e 1-e 0+e],c3);plot([0 1 1 0 0],[0 0 1 1 0]);
  Layer 1 : Subsystem
      mask Display: e = 0.06;c1 = [120 150 230]/255;c2 = ([120 150 230]+0)/383;c3 = ([120 150 230]+128)/383;patch([0 1 1 0],[0 0 1 1],c1);patch([0 1 1 1-e 1-e 0+e],[0 0 1 1-e 0+e 0+e],c2);patch([0 0 1 1-e 0+e 0+e],[0 1 1 1-e 1-e 0+e],c3);plot([0 1 1 0 0],[0 0 1 1 0]);
  Layer 2 : Subsystem
      mask Display: e = 0.06;c1 = [120 150 230]/255;c2 = ([120 150 230]+0)/383;c3 = ([120 150 230]+128)/383;patch([0 1 1 0],[0 0 1 1],c1);patch([0 1 1 1-e 1-e 0+e],[0 0 1 1-e 0+e 0+e],c2);patch([0 0 1 1-e 0+e 0+e],[0 1 1 1-e 1-e 0+e],c3);plot([0 1 1 0 0],[0 0 1 1 0]);
  Layer 3 : Subsystem
      mask Display: e = 0.06;c1 = [120 150 230]/255;c2 = ([120 150 230]+0)/383;c3 = ([120 150 230]+128)/383;patch([0 1 1 0],[0 0 1 1],c1);patch([0 1 1 1-e 1-e 0+e],[0 0 1 1-e 0+e 0+e],c2);patch([0 0 1 1-e 0+e 0+e],[0 1 1 1-e 1-e 0+e],c3);plot([0 1 1 0 0],[0 0 1 1 0]);
  Process Input 1 : Subsystem
      mask Display: e = 0.06;c1 = [120 150 230]/255;c2 = ([120 150 230]+0)/383;c3 = ([120 150 230]+128)/383;patch([0 1 1 0],[0 0 1 1],c1);patch([0 1 1 1-e 1-e 0+e],[0 0 1 1-e 0+e 0+e],c2);patch([0 0 1 1-e 0+e 0+e],[0 1 1 1-e 1-e 0+e],c3);plot([0 1 1 0 0],[0 0 1 1 0]);
  Process Output 1 : Subsystem
      mask Display: e = 0.06;c1 = [120 150 230]/255;c2 = ([120 150 230]+0)/383;c3 = ([120 150 230]+128)/383;patch([0 1 1 0],[0 0 1 1],c1);patch([0 1 1 1-e 1-e 0+e],[0 0 1 1-e 0+e 0+e],c2);patch([0 0 1 1-e 0+e 0+e],[0 1 1 1-e 1-e 0+e],c3);plot([0 1 1 0 0],[0 0 1 1 0]);
  Output : Outport  InitialOutput=[0;0]
      mask Display: e = 0.06;c1 = [120 230 180]/255;c2 = ([120 230 180]+0)/(256+128);c3 = ([120 230 180]+128)/(256+128);patch([0 1 1 0],[0 0 1 1],c1);patch([0 1 1 1-e 1-e 0+e],[0 0 1 1-e 0+e 0+e],c2);patch([0 0 1 1-e 0+e 0+e],[0 1 1 1-e 1-e 0+e],c3);plot([0 1 1 0 0],[0 0 1 1 0]);
  wires:
  Input/1 -> Process Input 1/1(x)
  Process Output 1/1(y) -> Output/1
  Process Input 1/1(p) -> Layer 1/1("p{1}")
  Layer 1/1("a{1}") -> [feedback1]
  [feedback1] -> Layer 2/1("a{1} ")
  Layer 2/1("a{2}") -> [feedback2]
  [feedback2] -> Layer 3/1("a{2} ")
  Layer 3/1("a{3}") -> Process Output 1/1(a)

== system MCVT_Integration/Sys2 - Structural System/Sys2_Structural_System/Fault Detection and Diagnosis (FDD) with Binary Classifer/Pattern Recognition Neural Network/Segment 3 ==
  Input : Inport  PortDimensions=2304
      mask Display: e = 0.06;c1 = [120 230 180]/255;c2 = ([120 230 180]+0)/(256+128);c3 = ([120 230 180]+128)/(256+128);patch([0 1 1 0],[0 0 1 1],c1);patch([0 1 1 1-e 1-e 0+e],[0 0 1 1-e 0+e 0+e],c2);patch([0 0 1 1-e 0+e 0+e],[0 1 1 1-e 1-e 0+e],c3);plot([0 1 1 0 0],[0 0 1 1 0]);
  Layer 1 : Subsystem
      mask Display: e = 0.06;c1 = [120 150 230]/255;c2 = ([120 150 230]+0)/383;c3 = ([120 150 230]+128)/383;patch([0 1 1 0],[0 0 1 1],c1);patch([0 1 1 1-e 1-e 0+e],[0 0 1 1-e 0+e 0+e],c2);patch([0 0 1 1-e 0+e 0+e],[0 1 1 1-e 1-e 0+e],c3);plot([0 1 1 0 0],[0 0 1 1 0]);
  Layer 2 : Subsystem
      mask Display: e = 0.06;c1 = [120 150 230]/255;c2 = ([120 150 230]+0)/383;c3 = ([120 150 230]+128)/383;patch([0 1 1 0],[0 0 1 1],c1);patch([0 1 1 1-e 1-e 0+e],[0 0 1 1-e 0+e 0+e],c2);patch([0 0 1 1-e 0+e 0+e],[0 1 1 1-e 1-e 0+e],c3);plot([0 1 1 0 0],[0 0 1 1 0]);
  Layer 3 : Subsystem
      mask Display: e = 0.06;c1 = [120 150 230]/255;c2 = ([120 150 230]+0)/383;c3 = ([120 150 230]+128)/383;patch([0 1 1 0],[0 0 1 1],c1);patch([0 1 1 1-e 1-e 0+e],[0 0 1 1-e 0+e 0+e],c2);patch([0 0 1 1-e 0+e 0+e],[0 1 1 1-e 1-e 0+e],c3);plot([0 1 1 0 0],[0 0 1 1 0]);
  Process Input 1 : Subsystem
      mask Display: e = 0.06;c1 = [120 150 230]/255;c2 = ([120 150 230]+0)/383;c3 = ([120 150 230]+128)/383;patch([0 1 1 0],[0 0 1 1],c1);patch([0 1 1 1-e 1-e 0+e],[0 0 1 1-e 0+e 0+e],c2);patch([0 0 1 1-e 0+e 0+e],[0 1 1 1-e 1-e 0+e],c3);plot([0 1 1 0 0],[0 0 1 1 0]);
  Process Output 1 : Subsystem
      mask Display: e = 0.06;c1 = [120 150 230]/255;c2 = ([120 150 230]+0)/383;c3 = ([120 150 230]+128)/383;patch([0 1 1 0],[0 0 1 1],c1);patch([0 1 1 1-e 1-e 0+e],[0 0 1 1-e 0+e 0+e],c2);patch([0 0 1 1-e 0+e 0+e],[0 1 1 1-e 1-e 0+e],c3);plot([0 1 1 0 0],[0 0 1 1 0]);
  Output : Outport  InitialOutput=[0;0]
      mask Display: e = 0.06;c1 = [120 230 180]/255;c2 = ([120 230 180]+0)/(256+128);c3 = ([120 230 180]+128)/(256+128);patch([0 1 1 0],[0 0 1 1],c1);patch([0 1 1 1-e 1-e 0+e],[0 0 1 1-e 0+e 0+e],c2);patch([0 0 1 1-e 0+e 0+e],[0 1 1 1-e 1-e 0+e],c3);plot([0 1 1 0 0],[0 0 1 1 0]);
  wires:
  Input/1 -> Process Input 1/1(x)
  Process Output 1/1(y) -> Output/1
  Process Input 1/1(p) -> Layer 1/1("p{1}")
  Layer 1/1("a{1}") -> [feedback1]
  [feedback1] -> Layer 2/1("a{1} ")
  Layer 2/1("a{2}") -> [feedback2]
  [feedback2] -> Layer 3/1("a{2} ")
  Layer 3/1("a{3}") -> Process Output 1/1(a)

== system MCVT_Integration/Sys2 - Structural System/Sys2_Structural_System/Fault Detection and Diagnosis (FDD) with Binary Classifer/Pattern Recognition Neural Network/Segment 4 ==
  Input : Inport  PortDimensions=2304
      mask Display: e = 0.06;c1 = [120 230 180]/255;c2 = ([120 230 180]+0)/(256+128);c3 = ([120 230 180]+128)/(256+128);patch([0 1 1 0],[0 0 1 1],c1);patch([0 1 1 1-e 1-e 0+e],[0 0 1 1-e 0+e 0+e],c2);patch([0 0 1 1-e 0+e 0+e],[0 1 1 1-e 1-e 0+e],c3);plot([0 1 1 0 0],[0 0 1 1 0]);
  Layer 1 : Subsystem
      mask Display: e = 0.06;c1 = [120 150 230]/255;c2 = ([120 150 230]+0)/383;c3 = ([120 150 230]+128)/383;patch([0 1 1 0],[0 0 1 1],c1);patch([0 1 1 1-e 1-e 0+e],[0 0 1 1-e 0+e 0+e],c2);patch([0 0 1 1-e 0+e 0+e],[0 1 1 1-e 1-e 0+e],c3);plot([0 1 1 0 0],[0 0 1 1 0]);
  Layer 2 : Subsystem
      mask Display: e = 0.06;c1 = [120 150 230]/255;c2 = ([120 150 230]+0)/383;c3 = ([120 150 230]+128)/383;patch([0 1 1 0],[0 0 1 1],c1);patch([0 1 1 1-e 1-e 0+e],[0 0 1 1-e 0+e 0+e],c2);patch([0 0 1 1-e 0+e 0+e],[0 1 1 1-e 1-e 0+e],c3);plot([0 1 1 0 0],[0 0 1 1 0]);
  Layer 3 : Subsystem
      mask Display: e = 0.06;c1 = [120 150 230]/255;c2 = ([120 150 230]+0)/383;c3 = ([120 150 230]+128)/383;patch([0 1 1 0],[0 0 1 1],c1);patch([0 1 1 1-e 1-e 0+e],[0 0 1 1-e 0+e 0+e],c2);patch([0 0 1 1-e 0+e 0+e],[0 1 1 1-e 1-e 0+e],c3);plot([0 1 1 0 0],[0 0 1 1 0]);
  Process Input 1 : Subsystem
      mask Display: e = 0.06;c1 = [120 150 230]/255;c2 = ([120 150 230]+0)/383;c3 = ([120 150 230]+128)/383;patch([0 1 1 0],[0 0 1 1],c1);patch([0 1 1 1-e 1-e 0+e],[0 0 1 1-e 0+e 0+e],c2);patch([0 0 1 1-e 0+e 0+e],[0 1 1 1-e 1-e 0+e],c3);plot([0 1 1 0 0],[0 0 1 1 0]);
  Process Output 1 : Subsystem
      mask Display: e = 0.06;c1 = [120 150 230]/255;c2 = ([120 150 230]+0)/383;c3 = ([120 150 230]+128)/383;patch([0 1 1 0],[0 0 1 1],c1);patch([0 1 1 1-e 1-e 0+e],[0 0 1 1-e 0+e 0+e],c2);patch([0 0 1 1-e 0+e 0+e],[0 1 1 1-e 1-e 0+e],c3);plot([0 1 1 0 0],[0 0 1 1 0]);
  Output : Outport  InitialOutput=[0;0]
      mask Display: e = 0.06;c1 = [120 230 180]/255;c2 = ([120 230 180]+0)/(256+128);c3 = ([120 230 180]+128)/(256+128);patch([0 1 1 0],[0 0 1 1],c1);patch([0 1 1 1-e 1-e 0+e],[0 0 1 1-e 0+e 0+e],c2);patch([0 0 1 1-e 0+e 0+e],[0 1 1 1-e 1-e 0+e],c3);plot([0 1 1 0 0],[0 0 1 1 0]);
  wires:
  Input/1 -> Process Input 1/1(x)
  Process Output 1/1(y) -> Output/1
  Process Input 1/1(p) -> Layer 1/1("p{1}")
  Layer 1/1("a{1}") -> [feedback1]
  [feedback1] -> Layer 2/1("a{1} ")
  Layer 2/1("a{2}") -> [feedback2]
  [feedback2] -> Layer 3/1("a{2} ")
  Layer 3/1("a{3}") -> Process Output 1/1(a)

== system MCVT_Integration/Sys2 - Structural System/Sys2_Structural_System/Fault Detection and Diagnosis (FDD) with Binary Classifer/Pattern Recognition Neural Network/Segment 5 ==
  Input : Inport  PortDimensions=2304
      mask Display: e = 0.06;c1 = [120 230 180]/255;c2 = ([120 230 180]+0)/(256+128);c3 = ([120 230 180]+128)/(256+128);patch([0 1 1 0],[0 0 1 1],c1);patch([0 1 1 1-e 1-e 0+e],[0 0 1 1-e 0+e 0+e],c2);patch([0 0 1 1-e 0+e 0+e],[0 1 1 1-e 1-e 0+e],c3);plot([0 1 1 0 0],[0 0 1 1 0]);
  Layer 1 : Subsystem
      mask Display: e = 0.06;c1 = [120 150 230]/255;c2 = ([120 150 230]+0)/383;c3 = ([120 150 230]+128)/383;patch([0 1 1 0],[0 0 1 1],c1);patch([0 1 1 1-e 1-e 0+e],[0 0 1 1-e 0+e 0+e],c2);patch([0 0 1 1-e 0+e 0+e],[0 1 1 1-e 1-e 0+e],c3);plot([0 1 1 0 0],[0 0 1 1 0]);
  Layer 2 : Subsystem
      mask Display: e = 0.06;c1 = [120 150 230]/255;c2 = ([120 150 230]+0)/383;c3 = ([120 150 230]+128)/383;patch([0 1 1 0],[0 0 1 1],c1);patch([0 1 1 1-e 1-e 0+e],[0 0 1 1-e 0+e 0+e],c2);patch([0 0 1 1-e 0+e 0+e],[0 1 1 1-e 1-e 0+e],c3);plot([0 1 1 0 0],[0 0 1 1 0]);
  Layer 3 : Subsystem
      mask Display: e = 0.06;c1 = [120 150 230]/255;c2 = ([120 150 230]+0)/383;c3 = ([120 150 230]+128)/383;patch([0 1 1 0],[0 0 1 1],c1);patch([0 1 1 1-e 1-e 0+e],[0 0 1 1-e 0+e 0+e],c2);patch([0 0 1 1-e 0+e 0+e],[0 1 1 1-e 1-e 0+e],c3);plot([0 1 1 0 0],[0 0 1 1 0]);
  Process Input 1 : Subsystem
      mask Display: e = 0.06;c1 = [120 150 230]/255;c2 = ([120 150 230]+0)/383;c3 = ([120 150 230]+128)/383;patch([0 1 1 0],[0 0 1 1],c1);patch([0 1 1 1-e 1-e 0+e],[0 0 1 1-e 0+e 0+e],c2);patch([0 0 1 1-e 0+e 0+e],[0 1 1 1-e 1-e 0+e],c3);plot([0 1 1 0 0],[0 0 1 1 0]);
  Process Output 1 : Subsystem
      mask Display: e = 0.06;c1 = [120 150 230]/255;c2 = ([120 150 230]+0)/383;c3 = ([120 150 230]+128)/383;patch([0 1 1 0],[0 0 1 1],c1);patch([0 1 1 1-e 1-e 0+e],[0 0 1 1-e 0+e 0+e],c2);patch([0 0 1 1-e 0+e 0+e],[0 1 1 1-e 1-e 0+e],c3);plot([0 1 1 0 0],[0 0 1 1 0]);
  Output : Outport  InitialOutput=[0;0]
      mask Display: e = 0.06;c1 = [120 230 180]/255;c2 = ([120 230 180]+0)/(256+128);c3 = ([120 230 180]+128)/(256+128);patch([0 1 1 0],[0 0 1 1],c1);patch([0 1 1 1-e 1-e 0+e],[0 0 1 1-e 0+e 0+e],c2);patch([0 0 1 1-e 0+e 0+e],[0 1 1 1-e 1-e 0+e],c3);plot([0 1 1 0 0],[0 0 1 1 0]);
  wires:
  Input/1 -> Process Input 1/1(x)
  Process Output 1/1(y) -> Output/1
  Process Input 1/1(p) -> Layer 1/1("p{1}")
  Layer 1/1("a{1}") -> [feedback1]
  [feedback1] -> Layer 2/1("a{1} ")
  Layer 2/1("a{2}") -> [feedback2]
  [feedback2] -> Layer 3/1("a{2} ")
  Layer 3/1("a{3}") -> Process Output 1/1(a)

== system MCVT_Integration/Sys2 - Structural System/Sys2_Structural_System/Fault Detection and Diagnosis (FDD) with Binary Classifer/Subsystem/Enabled Subsystem3 ==
  image : Inport
  thres : Inport  Port=2
  Enable : EnablePort
  MATLAB Function : MATLAB Function  TreatAsAtomicUnit=on
  Pattern Recognition Neural Network1 : Subsystem
      mask help: Pattern Recognition Neural Network
      mask Display: e = 0.06;c1 = [120 150 230]/255;c2 = ([120 150 230]+0)/383;c3 = ([120 150 230]+128)/383;patch([0 1 1 0],[0 0 1 1],c1);patch([0 1 1 1-e 1-e 0+e],[0 0 1 1-e 0+e 0+e],c2);patch([0 0 1 1-e 0+e 0+e],[0 1 1 1-e 1-e 0+e],c3);plot([0 1 1 0 0],[0 0 1 1 0]);fprintf('NNET');port_label('input',1,' Input');port_label('output',1,'Output ');
  y : Outport  OutputWhenDisabled=reset  InitialOutput=0
  wires:
  image/1 -> MATLAB Function/1(u)
  Pattern Recognition Neural Network1/1(Output) -> y/1
  MATLAB Function/1(y) -> Pattern Recognition Neural Network1/1(Input)
  thres/1 -> MATLAB Function/2(thres)

== system MCVT_Integration/Sys2 - Structural System/Sys2_Structural_System/Structural Thermal Model 2.2/STM Temperature Sensors/ Resistance Temperature Detector ==
  Physical Interdependency - IN : Inport
  Switch Control : Inport  Port=2
  Power_Supply (kW) : Inport  Port=3
  Power Consumption : MATLAB Function  TreatAsAtomicUnit=on
  R = U/I : Gain  Gain=1/0.001
  R vs T : MATLAB Function  TreatAsAtomicUnit=on
  RTD with Self-heat Error : Subsystem
  RTD without Self-heat Error : Subsystem
  Self-heat Calculation : Subsystem
  Switch ON/OFF Control Block : Subsystem
  T vs R : MATLAB Function  TreatAsAtomicUnit=on
  Physical Interdependency - OUT : Outport  VectorParamsAs1DForOutWhenUnconnected=off
  Cyber Interdependency - OUT : Outport  Port=2  VectorParamsAs1DForOutWhenUnconnected=off
  Power_Usage (kW) : Outport  Port=3
  wires:
  T vs R/1(R) -> RTD without Self-heat Error/1(Resistance Change without Error), Self-heat Calculation/2(Resistance Change without Error)
  R vs T/1(T) -> Cyber Interdependency - OUT/1
  RTD with Self-heat Error/1(Voltage Output with Error) -> R = U/I/1
  Self-heat Calculation/1(Resistance Change with Error) -> RTD with Self-heat Error/1(Resistance Change with Error)
  R = U/I/1 -> R vs T/1(R)
  RTD without Self-heat Error/1(Current in Circuit) -> Self-heat Calculation/3(Current in Circuit)
  Switch ON/OFF Control Block/1(Out1) -> Power Consumption/2(Switch), T vs R/1(T), Self-heat Calculation/1(Real Temperature)
  Switch Control/1 -> Switch ON/OFF Control Block/1(In1)
  Physical Interdependency - IN/1 -> Physical Interdependency - OUT/1, Switch ON/OFF Control Block/2(In2)
  Power_Supply (kW)/1 -> Power Consumption/1(Supply)
  Power Consumption/1(kWUsage1) -> Power_Usage (kW)/1

== system MCVT_Integration/Sys2 - Structural System/Sys2_Structural_System/Structural Thermal Model 2.2/STM Temperature Sensors/ Resistance Temperature Detector1 ==
  Physical Interdependency - IN : Inport
  Switch Control : Inport  Port=2
  Power_Supply (kW) : Inport  Port=3
  Power Consumption : MATLAB Function  TreatAsAtomicUnit=on
  R = U/I : Gain  Gain=1/0.001
  R vs T : MATLAB Function  TreatAsAtomicUnit=on
  RTD with Self-heat Error : Subsystem
  RTD without Self-heat Error : Subsystem
  Self-heat Calculation : Subsystem
  Switch ON/OFF Control Block : Subsystem
  T vs R : MATLAB Function  TreatAsAtomicUnit=on
  Physical Interdependency - OUT : Outport  VectorParamsAs1DForOutWhenUnconnected=off
  Cyber Interdependency - OUT : Outport  Port=2  VectorParamsAs1DForOutWhenUnconnected=off
  Power_Usage (kW) : Outport  Port=3
  wires:
  Physical Interdependency - IN/1 -> Switch ON/OFF Control Block/2(In2), Physical Interdependency - OUT/1
  Switch Control/1 -> Switch ON/OFF Control Block/1(In1)
  RTD without Self-heat Error/1(Current in Circuit) -> Self-heat Calculation/3(Current in Circuit)
  R = U/I/1 -> R vs T/1(R)
  Self-heat Calculation/1(Resistance Change with Error) -> RTD with Self-heat Error/1(Resistance Change with Error)
  RTD with Self-heat Error/1(Voltage Output with Error) -> R = U/I/1
  R vs T/1(T) -> Cyber Interdependency - OUT/1
  T vs R/1(R) -> Self-heat Calculation/2(Resistance Change without Error), RTD without Self-heat Error/1(Resistance Change without Error)
  Power Consumption/1(kWUsage2) -> Power_Usage (kW)/1
  Switch ON/OFF Control Block/1(Out1) -> Power Consumption/2(Switch), Self-heat Calculation/1(Real Temperature), T vs R/1(T)
  Power_Supply (kW)/1 -> Power Consumption/1(Supply)
